simulink model "AcasXuClosedLoop_all_comp2017b"  (Simulink 9.0)
settings: Solver=VariableStepAuto  StartTime=0.0  StopTime=20  RelTol=1e-3
libraries: neural x14040, simulink x2
(part 14 of 534)

== system AcasXuClosedLoop_all_comp2017b/networks/Networks_m_3/AcasXu_1_3/Process Input 1 ==
  x : Inport  PortDimensions=5
      mask Display: e = 0.06;c1 = [120 230 180]/255;c2 = ([120 230 180]+0)/(256+128);c3 = ([120 230 180]+128)/(256+128);patch([0 1 1 0],[0 0 1 1],c1);patch([0 1 1 1-e 1-e 0+e],[0 0 1 1-e 0+e 0+e],c2);patch([0 0 1 1-e 0+e 0+e],[0 1 1 1-e 1-e 0+e],c3);plot([0 1 1 0 0],[0 0 1 1 0]);
  p : Outport  PortDimensions=5
      mask Display: e = 0.06;c1 = [120 230 180]/255;c2 = ([120 230 180]+0)/(256+128);c3 = ([120 230 180]+128)/(256+128);patch([0 1 1 0],[0 0 1 1],c1);patch([0 1 1 1-e 1-e 0+e],[0 0 1 1-e 0+e 0+e],c2);patch([0 0 1 1-e 0+e 0+e],[0 1 1 1-e 1-e 0+e],c3);plot([0 1 1 0 0],[0 0 1 1 0]);
  wires:
  x/1 -> p/1

== system AcasXuClosedLoop_all_comp2017b/networks/Networks_m_3/AcasXu_1_3/Process Output 1 ==
  a : Inport  PortDimensions=5
      mask Display: e = 0.06;c1 = [120 230 180]/255;c2 = ([120 230 180]+0)/(256+128);c3 = ([120 230 180]+128)/(256+128);patch([0 1 1 0],[0 0 1 1],c1);patch([0 1 1 1-e 1-e 0+e],[0 0 1 1-e 0+e 0+e],c2);patch([0 0 1 1-e 0+e 0+e],[0 1 1 1-e 1-e 0+e],c3);plot([0 1 1 0 0],[0 0 1 1 0]);
  y : Outport  PortDimensions=5
      mask Display: e = 0.06;c1 = [120 230 180]/255;c2 = ([120 230 180]+0)/(256+128);c3 = ([120 230 180]+128)/(256+128);patch([0 1 1 0],[0 0 1 1],c1);patch([0 1 1 1-e 1-e 0+e],[0 0 1 1-e 0+e 0+e],c2);patch([0 0 1 1-e 0+e 0+e],[0 1 1 1-e 1-e 0+e],c3);plot([0 1 1 0 0],[0 0 1 1 0]);
  wires:
  a/1 -> y/1

== system AcasXuClosedLoop_all_comp2017b/networks/Networks_m_3/AcasXu_2_3/Layer 1 ==
  "p{1}" : Inport  PortDimensions=5
      mask Display: e = 0.06;c1 = [120 230 180]/255;c2 = ([120 230 180]+0)/(256+128);c3 = ([120 230 180]+128)/(256+128);patch([0 1 1 0],[0 0 1 1],c1);patch([0 1 1 1-e 1-e 0+e],[0 0 1 1-e 0+e 0+e],c2);patch([0 0 1 1-e 0+e 0+e],[0 1 1 1-e 1-e 0+e],c3);plot([0 1 1 0 0],[0 0 1 1 0]);
  Delays 1 : Subsystem
      mask Display: e = 0.06;c1 = [120 150 230]/255;c2 = ([120 150 230]+0)/383;c3 = ([120 150 230]+128)/383;patch([0 1 1 0],[0 0 1 1],c1);patch([0 1 1 1-e 1-e 0+e],[0 0 1 1-e 0+e 0+e],c2);patch([0 0 1 1-e 0+e 0+e],[0 1 1 1-e 1-e 0+e],c3);plot([0 1 1 0 0],[0 0 1 1 0]);disp('0');
  "IW{1,1}" : Subsystem
      mask Display: e = 0.06;c1 = [120 150 230]/255;c2 = ([120 150 230]+0)/383;c3 = ([120 150 230]+128)/383;patch([0 1 1 0],[0 0 1 1],c1);patch([0 1 1 1-e 1-e 0+e],[0 0 1 1-e 0+e 0+e],c2);patch([0 0 1 1-e 0+e 0+e],[0 1 1 1-e 1-e 0+e],c3);plot([0 1 1 0 0],[0 0 1 1 0]);disp('W');
  "b{1}" : Constant  Value=[-0.421252000000000015322854096666560508310794830322265625;0.2480300000000000004707345624410663731396198272705078125;0.06646539999999999392965577271752408705651760101318359375;-0.744869000000000003325340003357268869876861572265625;-0.040926099999999999978772535769167006947100162506103515625;0.01387269999999999996187494133437212440185248851776123046875;-0.258448000000000011056044968427158892154693603515625;-0.08566010000000000290665269631062983535230159759521484375;-0.1061060000000000058673066405390272848308086395263671875;-0.276390999999999997793764805464888922870159149169921875;0.20052700000000001079314415619592182338237762451171875;-0.1299849999999999894395585897655109874904155731201171875;-0.432665000000000021795898419441073201596736907958984375;-0.358543000000000000593303184359683655202388763427734375;-0.66513900000000003576161589080584235489368438720703125;-0.16696400000000000130029320644098334014415740966796875;-0.292223999999999983767651201560511253774166107177734375;-0.7251419999999999532747096964158117771148681640625;0.1654809999999999892139612711616791784763336181640625;-0.62143300000000001315214603891945444047451019287109375;-0.61700999999999994738431041696458123624324798583984375;-0.5631150000000000321875859299325384199619293212890625;0.04512449999999999794564331523361033760011196136474609375;-0.362211000000000005183409257369930855929851531982421875;-0.1272830000000000072457595479136216454207897186279296875;-0.2890989999999999948698814478120766580104827880859375;0.14068300000000000249400500251795165240764617919921875;-0.1167060000000000041797676431087893433868885040283203125;-0.0303211000000000001353139822413140791468322277069091796875;0.00203917999999999981219911404650702024810016155242919921875;-0.9795890000000000430446789323468692600727081298828125;-0.559147999999999978371079123462550342082977294921875;0.1838289999999999924096272252427297644317150115966796875;0.035959600000000001285282991148051223717629909515380859375;0.03314319999999999766249203503321041353046894073486328125;-0.056338199999999998002220280568508314900100231170654296875;0.11221499999999999530597705188483814708888530731201171875;-0.6100659999999999971720399116748012602329254150390625;-0.133693000000000006277645070440485142171382904052734375;-0.389712000000000002852829084076802246272563934326171875;0.2299690000000000067448269192027510143816471099853515625;-0.5140670000000000516848785991896875202655792236328125;-0.486846999999999974217956832944764755666255950927734375;0.06926279999999999936743932948957080952823162078857421875;0.047819699999999999706634667973048635758459568023681640625;-0.367711999999999983312903850674047134816646575927734375;-0.01804909999999999847819509568580542691051959991455078125;-0.56360600000000005138645065017044544219970703125;0.028249799999999998523225741564601776190102100372314453125;-0.2096550000000000080202511298921308480203151702880859375]
      mask Display: e = 0.06;c1 = [120 150 230]/255;c2 = ([120 150 230]+0)/383;c3 = ([120 150 230]+128)/383;patch([0 1 1 0],[0 0 1 1],c1);patch([0 1 1 1-e 1-e 0+e],[0 0 1 1-e 0+e 0+e],c2);patch([0 0 1 1-e 0+e 0+e],[0 1 1 1-e 1-e 0+e],c3);plot([0 1 1 0 0],[0 0 1 1 0]);disp('b');
  netsum : Sum  Inputs=2  SaturateOnIntegerOverflow=on  InputSameDT=on  OutDataTypeStr=Inherit: Same as first input
      mask Display: e = 0.06;c1 = [120 150 230]/255;c2 = ([120 150 230]+0)/383;c3 = ([120 150 230]+128)/383;patch([0 1 1 0],[0 0 1 1],c1);patch([0 1 1 1-e 1-e 0+e],[0 0 1 1-e 0+e 0+e],c2);patch([0 0 1 1-e 0+e 0+e],[0 1 1 1-e 1-e 0+e],c3);plot([0 1 1 0 0],[0 0 1 1 0]);disp('+');
  poslin : neural/Transfer Functions/poslin
      settings: SourceProductName=Deep Learning Toolbox  SourceProductBaseCode=NN
  "a{1}" : Outport  InitialOutput=[0;0;0;0;0;0;0;0;0;0;0;0;0;0;0;0;0;0;0;0;0;0;0;0;0;0;0;0;0;0;0;0;0;0;0;0;0;0;0;0;0;0;0;0;0;0;0;0;0;0]
      mask Display: e = 0.06;c1 = [120 230 180]/255;c2 = ([120 230 180]+0)/(256+128);c3 = ([120 230 180]+128)/(256+128);patch([0 1 1 0],[0 0 1 1],c1);patch([0 1 1 1-e 1-e 0+e],[0 0 1 1-e 0+e 0+e],c2);patch([0 0 1 1-e 0+e 0+e],[0 1 1 1-e 1-e 0+e],c3);plot([0 1 1 0 0],[0 0 1 1 0]);
  wires:
  poslin/1 -> "a{1}"/1
  netsum/1 -> poslin/1
  "b{1}"/1 -> netsum/2
  "IW{1,1}"/1("iz{1,1}") -> netsum/1
  Delays 1/1("pd{1,1}") -> "IW{1,1}"/1("pd{1,1}")
  "p{1}"/1 -> Delays 1/1("p{1}")

== system AcasXuClosedLoop_all_comp2017b/networks/Networks_m_3/AcasXu_2_3/Layer 2 ==
  "a{1} " : Inport  PortDimensions=50
      mask Display: e = 0.06;c1 = [120 230 180]/255;c2 = ([120 230 180]+0)/(256+128);c3 = ([120 230 180]+128)/(256+128);patch([0 1 1 0],[0 0 1 1],c1);patch([0 1 1 1-e 1-e 0+e],[0 0 1 1-e 0+e 0+e],c2);patch([0 0 1 1-e 0+e 0+e],[0 1 1 1-e 1-e 0+e],c3);plot([0 1 1 0 0],[0 0 1 1 0]);
  Delays 1 : Subsystem
      mask Display: e = 0.06;c1 = [120 150 230]/255;c2 = ([120 150 230]+0)/383;c3 = ([120 150 230]+128)/383;patch([0 1 1 0],[0 0 1 1],c1);patch([0 1 1 1-e 1-e 0+e],[0 0 1 1-e 0+e 0+e],c2);patch([0 0 1 1-e 0+e 0+e],[0 1 1 1-e 1-e 0+e],c3);plot([0 1 1 0 0],[0 0 1 1 0]);disp('0');
  "LW{2,1}" : Subsystem
      mask Display: e = 0.06;c1 = [120 150 230]/255;c2 = ([120 150 230]+0)/383;c3 = ([120 150 230]+128)/383;patch([0 1 1 0],[0 0 1 1],c1);patch([0 1 1 1-e 1-e 0+e],[0 0 1 1-e 0+e 0+e],c2);patch([0 0 1 1-e 0+e 0+e],[0 1 1 1-e 1-e 0+e],c3);plot([0 1 1 0 0],[0 0 1 1 0]);disp('W');
  "b{2}" : Constant  Value=[-0.838821999999999956543206280912272632122039794921875;-0.44406400000000001426769813406281173229217529296875;0.74864699999999995139177144665154628455638885498046875;-0.262479999999999991100452234604745171964168548583984375;0.75424199999999996801847146343789063394069671630859375;0.7134869999999999823359075890039093792438507080078125;0.4903370000000000228368435273296199738979339599609375;0.79215899999999994651744827933725900948047637939453125;0.88875300000000001521271997262374497950077056884765625;0.459341999999999972548181403908529318869113922119140625;-0.17511799999999999588595755994901992380619049072265625;0.94995700000000005136513436809764243662357330322265625;-0.92881400000000002847855284926481544971466064453125;-0.47367700000000001470112920287647284567356109619140625;0.439303999999999972292386019034893251955509185791015625;-0.00998504000000000058179239204037003219127655029296875;-0.349077999999999999403144101961515843868255615234375;0.528564999999999951540985421161167323589324951171875;0.412138999999999977585929400447639636695384979248046875;0.6882129999999999636628444932284764945507049560546875;0.95840099999999994739852127167978323996067047119140625;-0.3369400000000000172661884789704345166683197021484375;-0.391604000000000007641887123099877499043941497802734375;-0.00917568000000000029425795133874999010004103183746337890625;-0.01954759999999999831032937436248175799846649169921875;-0.348621000000000014207302001523203216493129730224609375;-0.4533490000000000019753088054130785167217254638671875;-0.7695499999999999563016217507538385689258575439453125;-0.59345400000000003704059281517402268946170806884765625;-0.32294000000000000483169060316868126392364501953125;-0.92078599999999999337063627535826526582241058349609375;0.288748999999999977905673631539684720337390899658203125;-0.9520690000000000541291456102044321596622467041015625;-0.1048509999999999997566391130021656863391399383544921875;-1.1722699999999999231903302643331699073314666748046875;-1.0962199999999999722177790317800827324390411376953125;-1.4131000000000000227373675443232059478759765625;0.206749999999999989341858963598497211933135986328125;-0.297868999999999994887645016206079162657260894775390625;-1.11681000000000008043343768804334104061126708984375;-0.894662999999999986044940669671632349491119384765625;0.1581220000000000125428556430051685310900211334228515625;-0.618076999999999987522869560052640736103057861328125;-0.404633999999999993679722365413908846676349639892578125;-1.21882000000000001449507180950604379177093505859375;-0.60074600000000000221689333557151257991790771484375;-1.074920000000000097628571893437765538692474365234375;-0.6672190000000000065227823142777197062969207763671875;-0.53613399999999999945288209346472285687923431396484375;-0.45271899999999998254196498237433843314647674560546875]
      mask Display: e = 0.06;c1 = [120 150 230]/255;c2 = ([120 150 230]+0)/383;c3 = ([120 150 230]+128)/383;patch([0 1 1 0],[0 0 1 1],c1);patch([0 1 1 1-e 1-e 0+e],[0 0 1 1-e 0+e 0+e],c2);patch([0 0 1 1-e 0+e 0+e],[0 1 1 1-e 1-e 0+e],c3);plot([0 1 1 0 0],[0 0 1 1 0]);disp('b');
  netsum : Sum  Inputs=2  SaturateOnIntegerOverflow=on  InputSameDT=on  OutDataTypeStr=Inherit: Same as first input
      mask Display: e = 0.06;c1 = [120 150 230]/255;c2 = ([120 150 230]+0)/383;c3 = ([120 150 230]+128)/383;patch([0 1 1 0],[0 0 1 1],c1);patch([0 1 1 1-e 1-e 0+e],[0 0 1 1-e 0+e 0+e],c2);patch([0 0 1 1-e 0+e 0+e],[0 1 1 1-e 1-e 0+e],c3);plot([0 1 1 0 0],[0 0 1 1 0]);disp('+');
  poslin : neural/Transfer Functions/poslin
      settings: SourceProductName=Deep Learning Toolbox  SourceProductBaseCode=NN
  "a{2}" : Outport  InitialOutput=[0;0;0;0;0;0;0;0;0;0;0;0;0;0;0;0;0;0;0;0;0;0;0;0;0;0;0;0;0;0;0;0;0;0;0;0;0;0;0;0;0;0;0;0;0;0;0;0;0;0]
      mask Display: e = 0.06;c1 = [120 230 180]/255;c2 = ([120 230 180]+0)/(256+128);c3 = ([120 230 180]+128)/(256+128);patch([0 1 1 0],[0 0 1 1],c1);patch([0 1 1 1-e 1-e 0+e],[0 0 1 1-e 0+e 0+e],c2);patch([0 0 1 1-e 0+e 0+e],[0 1 1 1-e 1-e 0+e],c3);plot([0 1 1 0 0],[0 0 1 1 0]);
  wires:
  poslin/1 -> "a{2}"/1
  netsum/1 -> poslin/1
  "b{2}"/1 -> netsum/2
  "LW{2,1}"/1("lz{2,1}") -> netsum/1
  Delays 1/1("pd{2,1}") -> "LW{2,1}"/1("ad{2,1}")
  "a{1} "/1 -> Delays 1/1("p{2}")

== system AcasXuClosedLoop_all_comp2017b/networks/Networks_m_3/AcasXu_2_3/Layer 3 ==
  "a{2} " : Inport  PortDimensions=50
      mask Display: e = 0.06;c1 = [120 230 180]/255;c2 = ([120 230 180]+0)/(256+128);c3 = ([120 230 180]+128)/(256+128);patch([0 1 1 0],[0 0 1 1],c1);patch([0 1 1 1-e 1-e 0+e],[0 0 1 1-e 0+e 0+e],c2);patch([0 0 1 1-e 0+e 0+e],[0 1 1 1-e 1-e 0+e],c3);plot([0 1 1 0 0],[0 0 1 1 0]);
  Delays 1 : Subsystem
      mask Display: e = 0.06;c1 = [120 150 230]/255;c2 = ([120 150 230]+0)/383;c3 = ([120 150 230]+128)/383;patch([0 1 1 0],[0 0 1 1],c1);patch([0 1 1 1-e 1-e 0+e],[0 0 1 1-e 0+e 0+e],c2);patch([0 0 1 1-e 0+e 0+e],[0 1 1 1-e 1-e 0+e],c3);plot([0 1 1 0 0],[0 0 1 1 0]);disp('0');
  "LW{3,2}" : Subsystem
      mask Display: e = 0.06;c1 = [120 150 230]/255;c2 = ([120 150 230]+0)/383;c3 = ([120 150 230]+128)/383;patch([0 1 1 0],[0 0 1 1],c1);patch([0 1 1 1-e 1-e 0+e],[0 0 1 1-e 0+e 0+e],c2);patch([0 0 1 1-e 0+e 0+e],[0 1 1 1-e 1-e 0+e],c3);plot([0 1 1 0 0],[0 0 1 1 0]);disp('W');
  "b{3}" : Constant  Value=[0.91208699999999998109245780142373405396938323974609375;-1.1068499999999998895106045893044210970401763916015625;0.1377939999999999998170352455417742021381855010986328125;-0.1684589999999999976321163330794661305844783782958984375;-1.424209999999999975983655531308613717555999755859375;0.78713800000000000434141611549421213567256927490234375;-0.12408500000000000085265128291212022304534912109375;1.3735999999999999321431687349104322493076324462890625;-0.00859632999999999924956028962697018869221210479736328125;-0.651855999999999990990318110561929643154144287109375;0.1454050000000000064659388954169116914272308349609375;0.06528219999999999856310495260913739912211894989013671875;-0.08414560000000000095088381613095407374203205108642578125;-0.011515799999999999647659620904960320331156253814697265625;-0.99867600000000000814992517916834913194179534912109375;0.2184359999999999912834169890629709698259830474853515625;0.50574500000000000010658141036401502788066864013671875;-0.56869300000000000405719902119017206132411956787109375;-0.024798699999999999910027526084377313964068889617919921875;-0.133927999999999991498356166630401276051998138427734375;-0.0165170000000000004314326673693358316086232662200927734375;-0.94237800000000004896349992122850380837917327880859375;-0.263867999999999991445065461448393762111663818359375;0.7654199999999999892708046900224871933460235595703125;-0.10988699999999999856736820902369800023734569549560546875;-0.82618000000000002547295707699959166347980499267578125;0.3943280000000000118376419777632690966129302978515625;-0.44726900000000002766142870314070023596286773681640625;0.298650999999999999801048033987171947956085205078125;-0.23718600000000000793676235844031907618045806884765625;0.54069299999999997918820326958666555583477020263671875;-0.71582800000000001983124775506439618766307830810546875;0.65939599999999998214406105034868232905864715576171875;-0.28665200000000001789857151379692368209362030029296875;-0.9983940000000000036806113712373189628124237060546875;-0.0237046999999999986885601543917800881899893283843994140625;-0.2134270000000000055973004009501892141997814178466796875;0.33173500000000000209610107049229554831981658935546875;-0.85767599999999999393907046396634541451930999755859375;-1.37675999999999998379962562466971576213836669921875;-0.954093999999999997640998117276467382907867431640625;-0.0253783999999999988761434366324465372599661350250244140625;0.0309684999999999995001775943137545255012810230255126953125;-0.74933199999999999807442918609012849628925323486328125;0.06617430000000000533955102355321287177503108978271484375;-0.035752499999999999558131236199187696911394596099853515625;-0.022454899999999999860023081055260263383388519287109375;-0.51581299999999996597210838444880209863185882568359375;-0.634611000000000036180836104904301464557647705078125;-0.265023999999999981813658678220235742628574371337890625]
      mask Display: e = 0.06;c1 = [120 150 230]/255;c2 = ([120 150 230]+0)/383;c3 = ([120 150 230]+128)/383;patch([0 1 1 0],[0 0 1 1],c1);patch([0 1 1 1-e 1-e 0+e],[0 0 1 1-e 0+e 0+e],c2);patch([0 0 1 1-e 0+e 0+e],[0 1 1 1-e 1-e 0+e],c3);plot([0 1 1 0 0],[0 0 1 1 0]);disp('b');
  netsum : Sum  Inputs=2  SaturateOnIntegerOverflow=on  InputSameDT=on  OutDataTypeStr=Inherit: Same as first input
      mask Display: e = 0.06;c1 = [120 150 230]/255;c2 = ([120 150 230]+0)/383;c3 = ([120 150 230]+128)/383;patch([0 1 1 0],[0 0 1 1],c1);patch([0 1 1 1-e 1-e 0+e],[0 0 1 1-e 0+e 0+e],c2);patch([0 0 1 1-e 0+e 0+e],[0 1 1 1-e 1-e 0+e],c3);plot([0 1 1 0 0],[0 0 1 1 0]);disp('+');
  poslin : neural/Transfer Functions/poslin
      settings: SourceProductName=Deep Learning Toolbox  SourceProductBaseCode=NN
  "a{3}" : Outport  InitialOutput=[0;0;0;0;0;0;0;0;0;0;0;0;0;0;0;0;0;0;0;0;0;0;0;0;0;0;0;0;0;0;0;0;0;0;0;0;0;0;0;0;0;0;0;0;0;0;0;0;0;0]
      mask Display: e = 0.06;c1 = [120 230 180]/255;c2 = ([120 230 180]+0)/(256+128);c3 = ([120 230 180]+128)/(256+128);patch([0 1 1 0],[0 0 1 1],c1);patch([0 1 1 1-e 1-e 0+e],[0 0 1 1-e 0+e 0+e],c2);patch([0 0 1 1-e 0+e 0+e],[0 1 1 1-e 1-e 0+e],c3);plot([0 1 1 0 0],[0 0 1 1 0]);
  wires:
  poslin/1 -> "a{3}"/1
  netsum/1 -> poslin/1
  "b{3}"/1 -> netsum/2
  "LW{3,2}"/1("lz{3,2}") -> netsum/1
  Delays 1/1("pd{3,2}") -> "LW{3,2}"/1("ad{3,2}")
  "a{2} "/1 -> Delays 1/1("p{3}")

== system AcasXuClosedLoop_all_comp2017b/networks/Networks_m_3/AcasXu_2_3/Layer 4 ==
  "a{3} " : Inport  PortDimensions=50
      mask Display: e = 0.06;c1 = [120 230 180]/255;c2 = ([120 230 180]+0)/(256+128);c3 = ([120 230 180]+128)/(256+128);patch([0 1 1 0],[0 0 1 1],c1);patch([0 1 1 1-e 1-e 0+e],[0 0 1 1-e 0+e 0+e],c2);patch([0 0 1 1-e 0+e 0+e],[0 1 1 1-e 1-e 0+e],c3);plot([0 1 1 0 0],[0 0 1 1 0]);
  Delays 1 : Subsystem
      mask Display: e = 0.06;c1 = [120 150 230]/255;c2 = ([120 150 230]+0)/383;c3 = ([120 150 230]+128)/383;patch([0 1 1 0],[0 0 1 1],c1);patch([0 1 1 1-e 1-e 0+e],[0 0 1 1-e 0+e 0+e],c2);patch([0 0 1 1-e 0+e 0+e],[0 1 1 1-e 1-e 0+e],c3);plot([0 1 1 0 0],[0 0 1 1 0]);disp('0');
  "LW{4,3}" : Subsystem
      mask Display: e = 0.06;c1 = [120 150 230]/255;c2 = ([120 150 230]+0)/383;c3 = ([120 150 230]+128)/383;patch([0 1 1 0],[0 0 1 1],c1);patch([0 1 1 1-e 1-e 0+e],[0 0 1 1-e 0+e 0+e],c2);patch([0 0 1 1-e 0+e 0+e],[0 1 1 1-e 1-e 0+e],c3);plot([0 1 1 0 0],[0 0 1 1 0]);disp('W');
  "b{4}" : Constant  Value=[0.3334449999999999914024328973027877509593963623046875;0.329369999999999996109778521713451482355594635009765625;0.404652999999999984925835860849474556744098663330078125;-0.9212839999999999918145476840436458587646484375;-0.329963999999999979539921923787915147840976715087890625;-1.8279399999999998982502802391536533832550048828125;-0.06095420000000000004813927034774678759276866912841796875;-2.620389999999999997015720509807579219341278076171875;1.2990500000000000380140363631653599441051483154296875;0.43092900000000000648725517748971469700336456298828125;-1.136530000000000040216718844021670520305633544921875;0.024272599999999998343636065101236454211175441741943359375;0.139058999999999988173016163273132406175136566162109375;-2.15068999999999999062083588796667754650115966796875;0.465926000000000006817657549618161283433437347412109375;-1.028140000000000053859139370615594089031219482421875;0.38497199999999998087929498069570399820804595947265625;-1.5580400000000000915179043659009039402008056640625;-0.042416399999999999936850514359321095980703830718994140625;-0.017387699999999998989874683275047573260962963104248046875;0.688755000000000006110667527536861598491668701171875;0.457880000000000009219291996487299911677837371826171875;-0.54451499999999997125854633850394748151302337646484375;0.1038010000000000043751668954428168945014476776123046875;-0.0096720799999999995666399854599148966372013092041015625;-0.1626849999999999962785324214564752764999866485595703125;-0.3657850000000000267874611381557770073413848876953125;-1.0444500000000001005417971100541763007640838623046875;2.10268999999999994798827174236066639423370361328125;-3.821079999999999810000872457749210298061370849609375;-4.04446999999999956543206280912272632122039794921875;-0.478206999999999993189447877739439718425273895263671875;-1.2907100000000000239452901951153762638568878173828125;0.633645000000000013784529073745943605899810791015625;-1.259980000000000099902308647870086133480072021484375;0.98899199999999998222932617863989435136318206787109375;-2.700790000000000024016344468691386282444000244140625;-0.032122499999999998276933865781757049262523651123046875;0.19799800000000000732569560568663291633129119873046875;0.671656000000000030780711313127540051937103271484375;-0.060253199999999999814104256756763788871467113494873046875;-1.59658999999999995367261362844146788120269775390625;-1.4014800000000000590461013416643254458904266357421875;-3.178430000000000088533624875708483159542083740234375;-1.257130000000000080717654782347381114959716796875;0.47790100000000002022915168709005229175090789794921875;-1.8170599999999998974686832298175431787967681884765625;1.0995299999999998963318148526013828814029693603515625;-1.1185099999999998932054268152569420635700225830078125;-1.21469000000000004746425474877469241619110107421875]
      mask Display: e = 0.06;c1 = [120 150 230]/255;c2 = ([120 150 230]+0)/383;c3 = ([120 150 230]+128)/383;patch([0 1 1 0],[0 0 1 1],c1);patch([0 1 1 1-e 1-e 0+e],[0 0 1 1-e 0+e 0+e],c2);patch([0 0 1 1-e 0+e 0+e],[0 1 1 1-e 1-e 0+e],c3);plot([0 1 1 0 0],[0 0 1 1 0]);disp('b');
  netsum : Sum  Inputs=2  SaturateOnIntegerOverflow=on  InputSameDT=on  OutDataTypeStr=Inherit: Same as first input
      mask Display: e = 0.06;c1 = [120 150 230]/255;c2 = ([120 150 230]+0)/383;c3 = ([120 150 230]+128)/383;patch([0 1 1 0],[0 0 1 1],c1);patch([0 1 1 1-e 1-e 0+e],[0 0 1 1-e 0+e 0+e],c2);patch([0 0 1 1-e 0+e 0+e],[0 1 1 1-e 1-e 0+e],c3);plot([0 1 1 0 0],[0 0 1 1 0]);disp('+');
  poslin : neural/Transfer Functions/poslin
      settings: SourceProductName=Deep Learning Toolbox  SourceProductBaseCode=NN
  "a{4}" : Outport  InitialOutput=[0;0;0;0;0;0;0;0;0;0;0;0;0;0;0;0;0;0;0;0;0;0;0;0;0;0;0;0;0;0;0;0;0;0;0;0;0;0;0;0;0;0;0;0;0;0;0;0;0;0]
      mask Display: e = 0.06;c1 = [120 230 180]/255;c2 = ([120 230 180]+0)/(256+128);c3 = ([120 230 180]+128)/(256+128);patch([0 1 1 0],[0 0 1 1],c1);patch([0 1 1 1-e 1-e 0+e],[0 0 1 1-e 0+e 0+e],c2);patch([0 0 1 1-e 0+e 0+e],[0 1 1 1-e 1-e 0+e],c3);plot([0 1 1 0 0],[0 0 1 1 0]);
  wires:
  poslin/1 -> "a{4}"/1
  netsum/1 -> poslin/1
  "b{4}"/1 -> netsum/2
  "LW{4,3}"/1("lz{4,3}") -> netsum/1
  Delays 1/1("pd{4,3}") -> "LW{4,3}"/1("ad{4,3}")
  "a{3} "/1 -> Delays 1/1("p{4}")

== system AcasXuClosedLoop_all_comp2017b/networks/Networks_m_3/AcasXu_2_3/Layer 5 ==
  "a{4} " : Inport  PortDimensions=50
      mask Display: e = 0.06;c1 = [120 230 180]/255;c2 = ([120 230 180]+0)/(256+128);c3 = ([120 230 180]+128)/(256+128);patch([0 1 1 0],[0 0 1 1],c1);patch([0 1 1 1-e 1-e 0+e],[0 0 1 1-e 0+e 0+e],c2);patch([0 0 1 1-e 0+e 0+e],[0 1 1 1-e 1-e 0+e],c3);plot([0 1 1 0 0],[0 0 1 1 0]);
  Delays 1 : Subsystem
      mask Display: e = 0.06;c1 = [120 150 230]/255;c2 = ([120 150 230]+0)/383;c3 = ([120 150 230]+128)/383;patch([0 1 1 0],[0 0 1 1],c1);patch([0 1 1 1-e 1-e 0+e],[0 0 1 1-e 0+e 0+e],c2);patch([0 0 1 1-e 0+e 0+e],[0 1 1 1-e 1-e 0+e],c3);plot([0 1 1 0 0],[0 0 1 1 0]);disp('0');
  "LW{5,4}" : Subsystem
      mask Display: e = 0.06;c1 = [120 150 230]/255;c2 = ([120 150 230]+0)/383;c3 = ([120 150 230]+128)/383;patch([0 1 1 0],[0 0 1 1],c1);patch([0 1 1 1-e 1-e 0+e],[0 0 1 1-e 0+e 0+e],c2);patch([0 0 1 1-e 0+e 0+e],[0 1 1 1-e 1-e 0+e],c3);plot([0 1 1 0 0],[0 0 1 1 0]);disp('W');
  "b{5}" : Constant  Value=[-0.8137389999999999901092451182194054126739501953125;1.7203900000000000858335624798201024532318115234375;0.302292999999999978388842691856552846729755401611328125;1.2280699999999999949551465761032886803150177001953125;-2.23986000000000018417267710901796817779541015625;-1.117399999999999948840923025272786617279052734375;0.78366199999999996972377402926213108003139495849609375;0.66137100000000004218492222207714803516864776611328125;0.53447699999999997988453515063156373798847198486328125;-1.0321400000000000574118530494160950183868408203125;2.021220000000000016626700016786344349384307861328125;1.360079999999999955662133288569748401641845703125;0.35252899999999998126298805800615809857845306396484375;0.60845800000000005436362471300526522099971771240234375;1.1605499999999999705124764659558422863483428955078125;-0.1439680000000000126281207712963805533945560455322265625;0.60213200000000000056132876125047914683818817138671875;-0.0770681000000000004934719299853895790874958038330078125;0.261714999999999975432984911094536073505878448486328125;0.1324899999999999966604491419275291264057159423828125;0.372844000000000008743228363528032787144184112548828125;1.9803200000000000802202748673153109848499298095703125;-0.80286800000000002608402382975327782332897186279296875;-0.058148100000000001175148867105235694907605648040771484375;-0.70470299999999996831689941245713271200656890869140625;-0.63512500000000005062616992290713824331760406494140625;-0.149021999999999987807086654356680810451507568359375;0.40107999999999999207744849627488292753696441650390625;1.27892999999999990023979989928193390369415283203125;1.2263100000000000111555209514335729181766510009765625;-1.09027999999999991587174008600413799285888671875;0.7498599999999999710098563809879124164581298828125;0.949662000000000006139089236967265605926513671875;0.399548999999999987498000564301037229597568511962890625;0.83329100000000000392219590139575302600860595703125;-0.64116499999999998493649400188587605953216552734375;-0.73797199999999996133936974729294888675212860107421875;0.90293699999999998961897063054493628442287445068359375;0.99583100000000002172129143218626268208026885986328125;-0.997608000000000050278003982384689152240753173828125;-2.656350000000000211031192520749755203723907470703125;0.436614000000000002099653784171096049249172210693359375;0.190682999999999991391774756266386248171329498291015625;-1.204860000000000042064129956997931003570556640625;-0.93301999999999996049382389173842966556549072265625;-0.84462599999999998789945721000549383461475372314453125;-0.53773400000000004528288854999118484556674957275390625;1.46314000000000010714984455262310802936553955078125;0.378352000000000021628920876537449657917022705078125;0.7683370000000000477058392789331264793872833251953125]
      mask Display: e = 0.06;c1 = [120 150 230]/255;c2 = ([120 150 230]+0)/383;c3 = ([120 150 230]+128)/383;patch([0 1 1 0],[0 0 1 1],c1);patch([0 1 1 1-e 1-e 0+e],[0 0 1 1-e 0+e 0+e],c2);patch([0 0 1 1-e 0+e 0+e],[0 1 1 1-e 1-e 0+e],c3);plot([0 1 1 0 0],[0 0 1 1 0]);disp('b');
  netsum : Sum  Inputs=2  SaturateOnIntegerOverflow=on  InputSameDT=on  OutDataTypeStr=Inherit: Same as first input
      mask Display: e = 0.06;c1 = [120 150 230]/255;c2 = ([120 150 230]+0)/383;c3 = ([120 150 230]+128)/383;patch([0 1 1 0],[0 0 1 1],c1);patch([0 1 1 1-e 1-e 0+e],[0 0 1 1-e 0+e 0+e],c2);patch([0 0 1 1-e 0+e 0+e],[0 1 1 1-e 1-e 0+e],c3);plot([0 1 1 0 0],[0 0 1 1 0]);disp('+');
  poslin : neural/Transfer Functions/poslin
      settings: SourceProductName=Deep Learning Toolbox  SourceProductBaseCode=NN
  "a{5}" : Outport  InitialOutput=[0;0;0;0;0;0;0;0;0;0;0;0;0;0;0;0;0;0;0;0;0;0;0;0;0;0;0;0;0;0;0;0;0;0;0;0;0;0;0;0;0;0;0;0;0;0;0;0;0;0]
      mask Display: e = 0.06;c1 = [120 230 180]/255;c2 = ([120 230 180]+0)/(256+128);c3 = ([120 230 180]+128)/(256+128);patch([0 1 1 0],[0 0 1 1],c1);patch([0 1 1 1-e 1-e 0+e],[0 0 1 1-e 0+e 0+e],c2);patch([0 0 1 1-e 0+e 0+e],[0 1 1 1-e 1-e 0+e],c3);plot([0 1 1 0 0],[0 0 1 1 0]);
  wires:
  poslin/1 -> "a{5}"/1
  netsum/1 -> poslin/1
  "b{5}"/1 -> netsum/2
  "LW{5,4}"/1("lz{5,4}") -> netsum/1
  Delays 1/1("pd{5,4}") -> "LW{5,4}"/1("ad{5,4}")
  "a{4} "/1 -> Delays 1/1("p{5}")

== system AcasXuClosedLoop_all_comp2017b/networks/Networks_m_3/AcasXu_2_3/Layer 6 ==
  "a{5} " : Inport  PortDimensions=50
      mask Display: e = 0.06;c1 = [120 230 180]/255;c2 = ([120 230 180]+0)/(256+128);c3 = ([120 230 180]+128)/(256+128);patch([0 1 1 0],[0 0 1 1],c1);patch([0 1 1 1-e 1-e 0+e],[0 0 1 1-e 0+e 0+e],c2);patch([0 0 1 1-e 0+e 0+e],[0 1 1 1-e 1-e 0+e],c3);plot([0 1 1 0 0],[0 0 1 1 0]);
  Delays 1 : Subsystem
      mask Display: e = 0.06;c1 = [120 150 230]/255;c2 = ([120 150 230]+0)/383;c3 = ([120 150 230]+128)/383;patch([0 1 1 0],[0 0 1 1],c1);patch([0 1 1 1-e 1-e 0+e],[0 0 1 1-e 0+e 0+e],c2);patch([0 0 1 1-e 0+e 0+e],[0 1 1 1-e 1-e 0+e],c3);plot([0 1 1 0 0],[0 0 1 1 0]);disp('0');
  "LW{6,5}" : Subsystem
      mask Display: e = 0.06;c1 = [120 150 230]/255;c2 = ([120 150 230]+0)/383;c3 = ([120 150 230]+128)/383;patch([0 1 1 0],[0 0 1 1],c1);patch([0 1 1 1-e 1-e 0+e],[0 0 1 1-e 0+e 0+e],c2);patch([0 0 1 1-e 0+e 0+e],[0 1 1 1-e 1-e 0+e],c3);plot([0 1 1 0 0],[0 0 1 1 0]);disp('W');
  "b{6}" : Constant  Value=[0.79271999999999998021138480908120982348918914794921875;-0.4281530000000000057980287238024175167083740234375;-0.5671150000000000357402996087330393493175506591796875;0.462776000000000020673240896940114907920360565185546875;0.429848999999999981103115942460135556757450103759765625;0.7204829999999999845528009245754219591617584228515625;0.69445999999999996621369291460723616182804107666015625;0.420893000000000017113421790782012976706027984619140625;0.2767749999999999932498440102790482342243194580078125;-0.04502609999999999945696771419534343294799327850341796875;-0.2369489999999999929602978454568074084818363189697265625;0.205720999999999987206678042639396153390407562255859375;0.19668900000000000272137867796118371188640594482421875;-0.98315600000000002989253289342741481959819793701171875;0.336816999999999977521980554229230619966983795166015625;-0.320413999999999976608933138777501881122589111328125;0.5474200000000000176925141204264946281909942626953125;0.424341000000000023728574660708545707166194915771484375;-0.032836700000000003385469682370967348106205463409423828125;-1.2760499999999999065636302475468255579471588134765625;0.1341769999999999907203118709730915725231170654296875;-0.49603900000000000769517782828188501298427581787109375;-0.7939220000000000165840674526407383382320404052734375;-0.06754380000000000106030739743800950236618518829345703125;-1.541349999999999997868371792719699442386627197265625;1.0422000000000000152766688188421539962291717529296875;0.38569799999999998529887079712352715432643890380859375;0.51283299999999998330935113699524663388729095458984375;0.5229340000000000099333874459262005984783172607421875;0.442062000000000010491163493497879244387149810791015625;-0.15205699999999999771915781821007840335369110107421875;-0.032714600000000003399147630034349276684224605560302734375;0.4078939999999999788116156196338124573230743408203125;-0.25559100000000001262634441445698030292987823486328125;0.373435000000000016928680679484386928379535675048828125;0.1337939999999999962643215667412732727825641632080078125;-0.94745299999999998963318148526013828814029693603515625;0.449172999999999988940402317894040606915950775146484375;0.431082000000000020722978888443321920931339263916015625;0.135475000000000012079226507921703159809112548828125;-0.287403999999999992809307514107786118984222412109375;0.326842000000000021397994487415417097508907318115234375;0.50904599999999999848654397283098660409450531005859375;-0.48480000000000000870414851306122727692127227783203125;-0.181304999999999993942623177645145915448665618896484375;0.07821160000000000611652950510688242502510547637939453125;0.029248900000000001286881712303511449135839939117431640625;0.409646000000000010121681270902627147734165191650390625;0.316469999999999973550046661330270580947399139404296875;-0.476785000000000014352963262354023754596710205078125]
      mask Display: e = 0.06;c1 = [120 150 230]/255;c2 = ([120 150 230]+0)/383;c3 = ([120 150 230]+128)/383;patch([0 1 1 0],[0 0 1 1],c1);patch([0 1 1 1-e 1-e 0+e],[0 0 1 1-e 0+e 0+e],c2);patch([0 0 1 1-e 0+e 0+e],[0 1 1 1-e 1-e 0+e],c3);plot([0 1 1 0 0],[0 0 1 1 0]);disp('b');
  netsum : Sum  Inputs=2  SaturateOnIntegerOverflow=on  InputSameDT=on  OutDataTypeStr=Inherit: Same as first input
      mask Display: e = 0.06;c1 = [120 150 230]/255;c2 = ([120 150 230]+0)/383;c3 = ([120 150 230]+128)/383;patch([0 1 1 0],[0 0 1 1],c1);patch([0 1 1 1-e 1-e 0+e],[0 0 1 1-e 0+e 0+e],c2);patch([0 0 1 1-e 0+e 0+e],[0 1 1 1-e 1-e 0+e],c3);plot([0 1 1 0 0],[0 0 1 1 0]);disp('+');
  poslin : neural/Transfer Functions/poslin
      settings: SourceProductName=Deep Learning Toolbox  SourceProductBaseCode=NN
  "a{6}" : Outport  InitialOutput=[0;0;0;0;0;0;0;0;0;0;0;0;0;0;0;0;0;0;0;0;0;0;0;0;0;0;0;0;0;0;0;0;0;0;0;0;0;0;0;0;0;0;0;0;0;0;0;0;0;0]
      mask Display: e = 0.06;c1 = [120 230 180]/255;c2 = ([120 230 180]+0)/(256+128);c3 = ([120 230 180]+128)/(256+128);patch([0 1 1 0],[0 0 1 1],c1);patch([0 1 1 1-e 1-e 0+e],[0 0 1 1-e 0+e 0+e],c2);patch([0 0 1 1-e 0+e 0+e],[0 1 1 1-e 1-e 0+e],c3);plot([0 1 1 0 0],[0 0 1 1 0]);
  wires:
  poslin/1 -> "a{6}"/1
  netsum/1 -> poslin/1
  "b{6}"/1 -> netsum/2
  "LW{6,5}"/1("lz{6,5}") -> netsum/1
  Delays 1/1("pd{6,5}") -> "LW{6,5}"/1("ad{6,5}")
  "a{5} "/1 -> Delays 1/1("p{6}")

== system AcasXuClosedLoop_all_comp2017b/networks/Networks_m_3/AcasXu_2_3/Layer 7 ==
  "a{6} " : Inport  PortDimensions=50
      mask Display: e = 0.06;c1 = [120 230 180]/255;c2 = ([120 230 180]+0)/(256+128);c3 = ([120 230 180]+128)/(256+128);patch([0 1 1 0],[0 0 1 1],c1);patch([0 1 1 1-e 1-e 0+e],[0 0 1 1-e 0+e 0+e],c2);patch([0 0 1 1-e 0+e 0+e],[0 1 1 1-e 1-e 0+e],c3);plot([0 1 1 0 0],[0 0 1 1 0]);
  Delays 1 : Subsystem
      mask Display: e = 0.06;c1 = [120 150 230]/255;c2 = ([120 150 230]+0)/383;c3 = ([120 150 230]+128)/383;patch([0 1 1 0],[0 0 1 1],c1);patch([0 1 1 1-e 1-e 0+e],[0 0 1 1-e 0+e 0+e],c2);patch([0 0 1 1-e 0+e 0+e],[0 1 1 1-e 1-e 0+e],c3);plot([0 1 1 0 0],[0 0 1 1 0]);disp('0');
  "LW{7,6}" : Subsystem
      mask Display: e = 0.06;c1 = [120 150 230]/255;c2 = ([120 150 230]+0)/383;c3 = ([120 150 230]+128)/383;patch([0 1 1 0],[0 0 1 1],c1);patch([0 1 1 1-e 1-e 0+e],[0 0 1 1-e 0+e 0+e],c2);patch([0 0 1 1-e 0+e 0+e],[0 1 1 1-e 1-e 0+e],c3);plot([0 1 1 0 0],[0 0 1 1 0]);disp('W');
  "b{7}" : Constant  Value=[-0.0208681999999999999440003506379071041010320186614990234375;-0.01963499999999999967581487680945429019629955291748046875;0.0180677999999999984448439960260657244361937046051025390625;-0.017649800000000000210764738994839717634022235870361328125;0.01822390000000000120916610057975049130618572235107421875]
      mask Display: e = 0.06;c1 = [120 150 230]/255;c2 = ([120 150 230]+0)/383;c3 = ([120 150 230]+128)/383;patch([0 1 1 0],[0 0 1 1],c1);patch([0 1 1 1-e 1-e 0+e],[0 0 1 1-e 0+e 0+e],c2);patch([0 0 1 1-e 0+e 0+e],[0 1 1 1-e 1-e 0+e],c3);plot([0 1 1 0 0],[0 0 1 1 0]);disp('b');
  netsum : Sum  Inputs=2  SaturateOnIntegerOverflow=on  InputSameDT=on  OutDataTypeStr=Inherit: Same as first input
      mask Display: e = 0.06;c1 = [120 150 230]/255;c2 = ([120 150 230]+0)/383;c3 = ([120 150 230]+128)/383;patch([0 1 1 0],[0 0 1 1],c1);patch([0 1 1 1-e 1-e 0+e],[0 0 1 1-e 0+e 0+e],c2);patch([0 0 1 1-e 0+e 0+e],[0 1 1 1-e 1-e 0+e],c3);plot([0 1 1 0 0],[0 0 1 1 0]);disp('+');
  purelin : neural/Transfer Functions/purelin
      settings: SourceProductName=Deep Learning Toolbox
  "a{7}" : Outport  InitialOutput=[0;0;0;0;0]
      mask Display: e = 0.06;c1 = [120 230 180]/255;c2 = ([120 230 180]+0)/(256+128);c3 = ([120 230 180]+128)/(256+128);patch([0 1 1 0],[0 0 1 1],c1);patch([0 1 1 1-e 1-e 0+e],[0 0 1 1-e 0+e 0+e],c2);patch([0 0 1 1-e 0+e 0+e],[0 1 1 1-e 1-e 0+e],c3);plot([0 1 1 0 0],[0 0 1 1 0]);
  wires:
  purelin/1 -> "a{7}"/1
  netsum/1 -> purelin/1
  "b{7}"/1 -> netsum/2
  "LW{7,6}"/1("lz{7,6}") -> netsum/1
  Delays 1/1("pd{7,6}") -> "LW{7,6}"/1("ad{7,6}")
  "a{6} "/1 -> Delays 1/1("p{7}")

== system AcasXuClosedLoop_all_comp2017b/networks/Networks_m_3/AcasXu_2_3/Process Input 1 ==
  x : Inport  PortDimensions=5
      mask Display: e = 0.06;c1 = [120 230 180]/255;c2 = ([120 230 180]+0)/(256+128);c3 = ([120 230 180]+128)/(256+128);patch([0 1 1 0],[0 0 1 1],c1);patch([0 1 1 1-e 1-e 0+e],[0 0 1 1-e 0+e 0+e],c2);patch([0 0 1 1-e 0+e 0+e],[0 1 1 1-e 1-e 0+e],c3);plot([0 1 1 0 0],[0 0 1 1 0]);
  p : Outport  PortDimensions=5
      mask Display: e = 0.06;c1 = [120 230 180]/255;c2 = ([120 230 180]+0)/(256+128);c3 = ([120 230 180]+128)/(256+128);patch([0 1 1 0],[0 0 1 1],c1);patch([0 1 1 1-e 1-e 0+e],[0 0 1 1-e 0+e 0+e],c2);patch([0 0 1 1-e 0+e 0+e],[0 1 1 1-e 1-e 0+e],c3);plot([0 1 1 0 0],[0 0 1 1 0]);
  wires:
  x/1 -> p/1

== system AcasXuClosedLoop_all_comp2017b/networks/Networks_m_3/AcasXu_2_3/Process Output 1 ==
  a : Inport  PortDimensions=5
      mask Display: e = 0.06;c1 = [120 230 180]/255;c2 = ([120 230 180]+0)/(256+128);c3 = ([120 230 180]+128)/(256+128);patch([0 1 1 0],[0 0 1 1],c1);patch([0 1 1 1-e 1-e 0+e],[0 0 1 1-e 0+e 0+e],c2);patch([0 0 1 1-e 0+e 0+e],[0 1 1 1-e 1-e 0+e],c3);plot([0 1 1 0 0],[0 0 1 1 0]);
  y : Outport  PortDimensions=5
      mask Display: e = 0.06;c1 = [120 230 180]/255;c2 = ([120 230 180]+0)/(256+128);c3 = ([120 230 180]+128)/(256+128);patch([0 1 1 0],[0 0 1 1],c1);patch([0 1 1 1-e 1-e 0+e],[0 0 1 1-e 0+e 0+e],c2);patch([0 0 1 1-e 0+e 0+e],[0 1 1 1-e 1-e 0+e],c3);plot([0 1 1 0 0],[0 0 1 1 0]);
  wires:
  a/1 -> y/1

== system AcasXuClosedLoop_all_comp2017b/networks/Networks_m_3/AcasXu_3_3/Layer 1 ==
  "p{1}" : Inport  PortDimensions=5
      mask Display: e = 0.06;c1 = [120 230 180]/255;c2 = ([120 230 180]+0)/(256+128);c3 = ([120 230 180]+128)/(256+128);patch([0 1 1 0],[0 0 1 1],c1);patch([0 1 1 1-e 1-e 0+e],[0 0 1 1-e 0+e 0+e],c2);patch([0 0 1 1-e 0+e 0+e],[0 1 1 1-e 1-e 0+e],c3);plot([0 1 1 0 0],[0 0 1 1 0]);
  Delays 1 : Subsystem
      mask Display: e = 0.06;c1 = [120 150 230]/255;c2 = ([120 150 230]+0)/383;c3 = ([120 150 230]+128)/383;patch([0 1 1 0],[0 0 1 1],c1);patch([0 1 1 1-e 1-e 0+e],[0 0 1 1-e 0+e 0+e],c2);patch([0 0 1 1-e 0+e 0+e],[0 1 1 1-e 1-e 0+e],c3);plot([0 1 1 0 0],[0 0 1 1 0]);disp('0');
  "IW{1,1}" : Subsystem
      mask Display: e = 0.06;c1 = [120 150 230]/255;c2 = ([120 150 230]+0)/383;c3 = ([120 150 230]+128)/383;patch([0 1 1 0],[0 0 1 1],c1);patch([0 1 1 1-e 1-e 0+e],[0 0 1 1-e 0+e 0+e],c2);patch([0 0 1 1-e 0+e 0+e],[0 1 1 1-e 1-e 0+e],c3);plot([0 1 1 0 0],[0 0 1 1 0]);disp('W');
  "b{1}" : Constant  Value=[-0.497039999999999981827869532935437746345996856689453125;0.051020900000000000973354730149367242120206356048583984375;-0.33802399999999999113953208507155068218708038330078125;-0.603275000000000005684341886080801486968994140625;0.09330149999999999554933793888267246074974536895751953125;-0.480101999999999973223197002880624495446681976318359375;0.1474000000000000032418512319054570980370044708251953125;-0.14948200000000000375877107217092998325824737548828125;-0.01733490000000000030855318300382350571453571319580078125;-0.08105279999999999429594055300185573287308216094970703125;0.13344400000000000705568936609779484570026397705078125;-0.191929999999999989501731079144519753754138946533203125;-0.1900370000000000114681597551680169999599456787109375;0.5081480000000000440962821812718175351619720458984375;0.25924599999999997645971916426788084208965301513671875;-0.4318400000000000016342482922482304275035858154296875;0.06291940000000000032809310823722626082599163055419921875;-0.36622900000000002673772314665256999433040618896484375;0.07663209999999999466613331833286792971193790435791015625;-0.337851999999999985657694878682377748191356658935546875;0.356542999999999998816946344959433190524578094482421875;-0.26125100000000001099209612220874987542629241943359375;-0.020522800000000000764099894468017737381160259246826171875;-0.0188351999999999998591793115565451444126665592193603515625;-0.41659699999999999509014969589770771563053131103515625;-0.64070199999999999374011849795351736247539520263671875;0.1478810000000000124398269463199540041387081146240234375;-0.023231200000000000460698146298454958014190196990966796875;-0.486526000000000014011902749189175665378570556640625;-0.27305299999999999016608853708021342754364013671875;0.40787099999999998356514652186888270080089569091796875;-0.325931000000000026251001372656901367008686065673828125;-0.44453500000000001346478484265389852225780487060546875;-0.2217659999999999909103820527889183722436428070068359375;-0.10711600000000000287858625824810587801039218902587890625;-0.85363299999999997513810967575409449636936187744140625;-0.31786300000000000665068000671453773975372314453125;0.2052670000000000050111026439481065608561038970947265625;-0.0238779999999999999193978084122136351652443408966064453125;0.616819000000000006167510946397669613361358642578125;0.014796600000000000030286884111774270422756671905517578125;-0.15210000000000001296740492762182839214801788330078125;-0.046579799999999997595079292977970908395946025848388671875;-0.023838999999999999079847157190670259296894073486328125;0.38264399999999998414068613783456385135650634765625;-0.265257000000000020545343204503296874463558197021484375;-0.448097000000000023067769916451652534306049346923828125;-0.56933299999999997798028061879449523985385894775390625;0.0949498000000000008657963235236820764839649200439453125;0.08354620000000000101803010466028354130685329437255859375]
      mask Display: e = 0.06;c1 = [120 150 230]/255;c2 = ([120 150 230]+0)/383;c3 = ([120 150 230]+128)/383;patch([0 1 1 0],[0 0 1 1],c1);patch([0 1 1 1-e 1-e 0+e],[0 0 1 1-e 0+e 0+e],c2);patch([0 0 1 1-e 0+e 0+e],[0 1 1 1-e 1-e 0+e],c3);plot([0 1 1 0 0],[0 0 1 1 0]);disp('b');
  netsum : Sum  Inputs=2  SaturateOnIntegerOverflow=on  InputSameDT=on  OutDataTypeStr=Inherit: Same as first input
      mask Display: e = 0.06;c1 = [120 150 230]/255;c2 = ([120 150 230]+0)/383;c3 = ([120 150 230]+128)/383;patch([0 1 1 0],[0 0 1 1],c1);patch([0 1 1 1-e 1-e 0+e],[0 0 1 1-e 0+e 0+e],c2);patch([0 0 1 1-e 0+e 0+e],[0 1 1 1-e 1-e 0+e],c3);plot([0 1 1 0 0],[0 0 1 1 0]);disp('+');
  poslin : neural/Transfer Functions/poslin
      settings: SourceProductName=Deep Learning Toolbox  SourceProductBaseCode=NN
  "a{1}" : Outport  InitialOutput=[0;0;0;0;0;0;0;0;0;0;0;0;0;0;0;0;0;0;0;0;0;0;0;0;0;0;0;0;0;0;0;0;0;0;0;0;0;0;0;0;0;0;0;0;0;0;0;0;0;0]
      mask Display: e = 0.06;c1 = [120 230 180]/255;c2 = ([120 230 180]+0)/(256+128);c3 = ([120 230 180]+128)/(256+128);patch([0 1 1 0],[0 0 1 1],c1);patch([0 1 1 1-e 1-e 0+e],[0 0 1 1-e 0+e 0+e],c2);patch([0 0 1 1-e 0+e 0+e],[0 1 1 1-e 1-e 0+e],c3);plot([0 1 1 0 0],[0 0 1 1 0]);
  wires:
  poslin/1 -> "a{1}"/1
  netsum/1 -> poslin/1
  "b{1}"/1 -> netsum/2
  "IW{1,1}"/1("iz{1,1}") -> netsum/1
  Delays 1/1("pd{1,1}") -> "IW{1,1}"/1("pd{1,1}")
  "p{1}"/1 -> Delays 1/1("p{1}")

== system AcasXuClosedLoop_all_comp2017b/networks/Networks_m_3/AcasXu_3_3/Layer 2 ==
  "a{1} " : Inport  PortDimensions=50
      mask Display: e = 0.06;c1 = [120 230 180]/255;c2 = ([120 230 180]+0)/(256+128);c3 = ([120 230 180]+128)/(256+128);patch([0 1 1 0],[0 0 1 1],c1);patch([0 1 1 1-e 1-e 0+e],[0 0 1 1-e 0+e 0+e],c2);patch([0 0 1 1-e 0+e 0+e],[0 1 1 1-e 1-e 0+e],c3);plot([0 1 1 0 0],[0 0 1 1 0]);
  Delays 1 : Subsystem
      mask Display: e = 0.06;c1 = [120 150 230]/255;c2 = ([120 150 230]+0)/383;c3 = ([120 150 230]+128)/383;patch([0 1 1 0],[0 0 1 1],c1);patch([0 1 1 1-e 1-e 0+e],[0 0 1 1-e 0+e 0+e],c2);patch([0 0 1 1-e 0+e 0+e],[0 1 1 1-e 1-e 0+e],c3);plot([0 1 1 0 0],[0 0 1 1 0]);disp('0');
  "LW{2,1}" : Subsystem
      mask Display: e = 0.06;c1 = [120 150 230]/255;c2 = ([120 150 230]+0)/383;c3 = ([120 150 230]+128)/383;patch([0 1 1 0],[0 0 1 1],c1);patch([0 1 1 1-e 1-e 0+e],[0 0 1 1-e 0+e 0+e],c2);patch([0 0 1 1-e 0+e 0+e],[0 1 1 1-e 1-e 0+e],c3);plot([0 1 1 0 0],[0 0 1 1 0]);disp('W');
  "b{2}" : Constant  Value=[-0.1070770000000000055084825589801766909658908843994140625;-0.540758999999999989682919476763345301151275634765625;-0.6799180000000000223536744670127518475055694580078125;-0.0222550999999999998824051772317034192383289337158203125;0.4184550000000000213873363463790155947208404541015625;-0.393732000000000026407320774524123407900333404541015625;0.1176939999999999930668792558208224363625049591064453125;0.853423999999999960408558763447217643260955810546875;0.094516199999999994663113511705887503921985626220703125;-0.422592000000000023174351326815667562186717987060546875;-1.3403400000000000868993765834602527320384979248046875;-0.87682099999999996153832171330577693879604339599609375;0.606670999999999960294871925725601613521575927734375;-0.75119499999999994610533349259640090167522430419921875;-0.468569000000000013272938303998671472072601318359375;-1.0661400000000000876099193192203529179096221923828125;0.4733519999999999949835682855336926877498626708984375;0.280316999999999982851051072429982014000415802001953125;-0.82506299999999999084110413605230860412120819091796875;-0.264838999999999991086241379889543168246746063232421875;-1.038370000000000015205614545266143977642059326171875;-0.81917300000000004001066145065124146640300750732421875;-0.56666799999999994952304405160248279571533203125;-0.340478000000000002867039938792004249989986419677734375;0.28116799999999997350386138350586406886577606201171875;0.0002457909999999999782482884569390080287121236324310302734375;-0.0145389999999999998403499290589024894870817661285400390625;0.01712160000000000070752292913311976008117198944091796875;-0.60843100000000005511680001291097141802310943603515625;0.1356340000000000045599080067404429428279399871826171875;0.55756200000000000205346850634668953716754913330078125;-0.7212300000000000377298192688613198697566986083984375;0.20034899999999999931077354631270281970500946044921875;-1.140859999999999985220711096189916133880615234375;-0.77150799999999997158539599695359356701374053955078125;-0.4939270000000000049311665861750952899456024169921875;-0.42535299999999998110666865613893605768680572509765625;0.154399000000000008459011269223992712795734405517578125;-0.71662499999999995647925743469386361539363861083984375;-0.65694799999999997641708660012227483093738555908203125;0.0512156000000000000138555833473219536244869232177734375;0.1727059999999999984066079150579753331840038299560546875;-0.7631360000000000365361074727843515574932098388671875;-0.8705629999999999757420710011501796543598175048828125;-0.33361899999999999888444790485664270818233489990234375;-0.576794000000000028904878490720875561237335205078125;-0.0212333000000000000906386077303977799601852893829345703125;0.778575000000000017053025658242404460906982421875;-1.2952799999999999869260136620141565799713134765625;-0.69197100000000000274980038739158771932125091552734375]
      mask Display: e = 0.06;c1 = [120 150 230]/255;c2 = ([120 150 230]+0)/383;c3 = ([120 150 230]+128)/383;patch([0 1 1 0],[0 0 1 1],c1);patch([0 1 1 1-e 1-e 0+e],[0 0 1 1-e 0+e 0+e],c2);patch([0 0 1 1-e 0+e 0+e],[0 1 1 1-e 1-e 0+e],c3);plot([0 1 1 0 0],[0 0 1 1 0]);disp('b');
  netsum : Sum  Inputs=2  SaturateOnIntegerOverflow=on  InputSameDT=on  OutDataTypeStr=Inherit: Same as first input
      mask Display: e = 0.06;c1 = [120 150 230]/255;c2 = ([120 150 230]+0)/383;c3 = ([120 150 230]+128)/383;patch([0 1 1 0],[0 0 1 1],c1);patch([0 1 1 1-e 1-e 0+e],[0 0 1 1-e 0+e 0+e],c2);patch([0 0 1 1-e 0+e 0+e],[0 1 1 1-e 1-e 0+e],c3);plot([0 1 1 0 0],[0 0 1 1 0]);disp('+');
  poslin : neural/Transfer Functions/poslin
      settings: SourceProductName=Deep Learning Toolbox  SourceProductBaseCode=NN
  "a{2}" : Outport  InitialOutput=[0;0;0;0;0;0;0;0;0;0;0;0;0;0;0;0;0;0;0;0;0;0;0;0;0;0;0;0;0;0;0;0;0;0;0;0;0;0;0;0;0;0;0;0;0;0;0;0;0;0]
      mask Display: e = 0.06;c1 = [120 230 180]/255;c2 = ([120 230 180]+0)/(256+128);c3 = ([120 230 180]+128)/(256+128);patch([0 1 1 0],[0 0 1 1],c1);patch([0 1 1 1-e 1-e 0+e],[0 0 1 1-e 0+e 0+e],c2);patch([0 0 1 1-e 0+e 0+e],[0 1 1 1-e 1-e 0+e],c3);plot([0 1 1 0 0],[0 0 1 1 0]);
  wires:
  poslin/1 -> "a{2}"/1
  netsum/1 -> poslin/1
  "b{2}"/1 -> netsum/2
  "LW{2,1}"/1("lz{2,1}") -> netsum/1
  Delays 1/1("pd{2,1}") -> "LW{2,1}"/1("ad{2,1}")
  "a{1} "/1 -> Delays 1/1("p{2}")
